simulink model "PlanarModelandControl"  (R2026a)
settings: Solver=FixedStepDiscrete  StopTime=StopTime  FixedStep=Ts  MaxStep=0  RelTol=1e-5  AbsTol=1e-3
PostLoadFcn:
  | Controles_Ativos = 1;
  | Vx_Ref = 0;
  | Vx_init = 0;
  | t_default = [0; 10000];
  | Brake_Cmd = [t_default, [0; 0]];
  | Accel_Cmd = [t_default, [0; 0]];
  | Steer_Cmd = [t_default, [0; 0]];
  | Flag_CruiseControl = 0;
  | Ts = 0.001;
  | load('TC_LUT.mat')
InitFcn:
  | % Carrega parâmetros gerais (Massa, Ganhos PID, Geometria)
  | run('Parameters.m');
  | 
  | % Carrega as Tabelas (Lookup Tables)
  | run('curva_steering.m');
  | run('curva_tq_motor.m');
  | load('TC_LUT.mat')
model workspace (MAT data in the model file):
  A = 1.22
  Ack = 1
  Cd = 1.1
  Cl = -2
  Cs = [19075.58 25992.8 31792.64 35898.02 39044.37]
  Fz0x = -811.9083
  Fz0y = -210.9467
  Fz_cs = [222.41 444.82 667.23 889.64 1112.05]
  IAY = 0
  IAx = 0
  IAy = 0
  Im = 0.00033
  It = 0.0094
  Iw = 0.1578
  Iw_ = 0.7
  Iz_car = 180
  K_50_understeer = 0.005184
  K_Stability = 1
  K_oversteer = -0.001
  Kappa_F = 0
  Kappa_R = 0
  Km_FM = 110000
  Km_Fm = 110000
  Km_RM = 120000
  Km_Rm = 120000
  KphiARB_F = 0
  KphiARB_R = 11000
  KphiF = 17054.5
  KphiR = 40034.8
  Kphim_F = 137500
  Kphim_R = 48600
  L = 1.53
  M = 300
  Mgt = 11.72
  P = 68.9476
  P0 = 55.4852
  R = 0.2032
  Rcurva = 20
  Re = 0.2
  SR = 3
  TV_On = 1
  Ts = 0.001
  W = 2940
  Wf = 308.034
  Wr = 339.426
  Ws = 2099.34
  YLL = 1.11022302463e-16
  cx1 = 1.3668
  cy1 = 1.839
  dpi = 0.242630467224
  dx1 = 2.1568
  dx2 = -0.065814
  dx3 = 0.0011254
  dy1 = 2.453
  dy2 = -0.11197
  dy3 = 10.2899
  ex1 = -0.19815
  ex2 = -0.62529
  ex3 = 1.3396
  ex4 = 0.0029464
  ey1 = 0.96085
  ey2 = -0.28662
  ey3 = 0.14389
  ey4 = 4.9274
  ey5 = -70.7165
  g = 9.81
  hcg = 0.252
  hcgs = 0.202
  hrc_f = 0.056
  hrc_r = 0.084
  hs_f = 0.222
  hs_r = 0.222
  hx1 = -0.003859
  hx2 = -0.000374
  hy1 = -0.000403
  hy2 = -0.000261
  kx1 = -36.9039
  kx2 = 22.3857
  kx3 = 0.61844
  ky1 = -83.2526
  ky2 = -5.565
  ky3 = 0.88491
  ky4 = 2.9994
  ky5 = -99.9393
  ky6 = -3.4705
  ky7 = -0.91452
  lf = 0.8415
  lr = 0.6885
  mass_FL = 67.5
  mass_FR = 67.5
  mass_RL = 82.5
  mass_RR = 82.5
  mi = 2
  ms = 240
  pty1 = 0.005744
  pty2 = 19.1334
  px1 = -0.039964
  px2 = -0.15015
  px3 = -0.32528
  px4 = -0.063273
  py1 = 0.38578
  py2 = 1.2048
  py3 = -0.14544
  py4 = 0.092886
  py5 = -0.82901
  r = 9
  rhoar = 1.22
  s_f = 1
  s_r = 0.9
  tf = 1.25
  tr = 1.25
  vx1 = -0.11922
  vx2 = 0.002205
  vy1 = 0.032907
  vy2 = 0.018068
  vy3 = -1.0429
  vy4 = 0.53712
  x_fl = 0.8415
  x_fr = 0.8415
  x_rl = 0.6885
  x_rr = 0.6885
  xs_f = 0.826
  xs_r = 0.704
  y_fl = 0.6
  y_fr = 0.6
  y_rl = 0.59
  y_rr = 0.59
values from neighbouring files (symbols this model uses):
  GG_mode = 0    [autocross.m]    (also in teste.mat)
  Gr = 11.72    [Parameters.m]    (also in teste.mat)
  K_variavel = [-0.001, 0, 0.0008, 0.0012, 0.0015]    [Parameters.m]    (also in teste.mat = [-0.001 0 0.0008 0.0012 0.0015])
  Ki_data = [283117.9493, 114831.615, 51596.0519, 30556.5344, 17525.221, 11773.8301, 10567.5001]*0.008    [Parameters.m]    (also in teste.mat = [2264.9435944 918.65292 412.7684152 244.4522752 140.201768 9)
  Kp_data = [2877.5702, 2303.9999, 1972.0835, 1556.8775, 1258.2451, 1022.7085, 982.7861, ]*5    [Parameters.m]    (also in teste.mat = [14387.851 11519.9995 9860.4175 7784.3875 6291.2255 5113.542)
  Mz_maxF = [0 66.3 132.6 198.9 265.2 330.23 396.52 462.83 529.112 595.42 661.73 728.03 794.33 860.62 926.92 993.23 1058.25 1124.55 1190.85 1257.15 1323.45 1389.75 1456.05 1522.35 1588.65 1654.95]    [Parameters.m]    (also in teste.mat = [0 66.3 132.6 198.9 265.2 330.23 396.52 462.83 529.112 595.4)
  Mz_minF = [0 -66.3 -132.6 -198.9 -265.2 -330.23 -396.52 -462.83 -529.112 -595.42 -661.73 -728.03 -794.33 -860.62 -926.92 -993.23 -1058.25 -1124.55 -1190.85 -1257.15 -1323.45 -1389.75 -1456.05 -1522.35 -1588.65 -1654.95]    [Parameters.m]    (also in teste.mat = [0 -66.3 -132.6 -198.9 -265.2 -330.23 -396.52 -462.83 -529.1)
  pedal = [0 4 8 12 16 20 24 28 32 36 40 44 48 52 56 60 64 68 72 76 80 84 88 92 96 100]    [Parameters.m]    (also in teste.mat = [0 4 8 12 16 20 24 28 32 36 40 44 ... (26 elements, 1x26)])
  rpm_355_novo = [0 10050 10100 10140 10170 10210 10250 10280 10320 10360         10390 10420 10460 10620 10770 10920 11050 11170 11280         11380 11470 11550 11625 11680 11730 11775 11790 11800]    [curva_tq_motor.m]    (also in teste.mat = [0 10050 10100 10140 10170 10210 10250 10280 10320 10360 103)
  Steering_inner = [-29.018 ... (first row only)    [curva_steering.m]    (also in teste.mat = [-29.018 -25.595 -22.357 -19.265 -16.292 -13.415 -10.619 -7.)
  Steering_outer = [-25.111 ... (first row only)    [curva_steering.m]    (also in teste.mat = [-25.111 -22.589 -20.082 -17.585 -15.094 -12.604 -10.11 -7.6)
  steering_vol = [-105 ... (first row only)    [curva_steering.m]    (also in teste.mat = [-105 -94.5 -84 -73.5 -63 -52.5 -42 -31.5 -21 -10.5 0 10.5 .)
  torque_355_novo = [16.2 16.2 16.0 15.8 15.6 15.4 15.2 15.0 14.8 14.6            14.4 14.2 14.0 13.0 12.0 11.0 10.0 9.0 8.0            7.0 6.0 5.0 4.0 3.0 2.0 1.0 0.5 0.0]    [curva_tq_motor.m]    (also in teste.mat = [16.2 16.2 16 15.8 15.6 15.4 15.2 15 14.8 14.6 14.4 14.2 ...)
  Vx = [0, 12.5, 23.6, 30, 35]    [Parameters.m]    (also in teste.mat = [0 12.5 23.6 30 35])
  Vx_axis = [5, 10, 15, 20, 25, 30, 35]    [Parameters.m]    (also in teste.mat = [5 10 15 20 25 30 35])
undefined symbols (not defined in the file or in its folder): DiscIntNeverNeededParam, magnitude
libraries: simulink x5, fuzblock x4, sl_discontinuities x3, simulink_extras x3, slpidlib x1
(part 3 of 4)

== system PlanarModelandControl/Planta/Dinamica do Veículo/Load Transfer/Longitudinal Weight Transfer ==
  note: Transferencia de Carga - Longitudinal
  Bus Element In4 : Inport  PortName=Movimento  Element=Ax_cg
  Discrete Transfer Fcn1 : DiscreteTransferFcn  Numerator=[0.1813]  Denominator="[1  -0.8187]"
  Gain21 : Gain  Gain=-1/2*W*(1-2*YLL/tf)*(hcg/(L*g))
  Gain22 : Gain  Gain=1/2*W*hcg*(1-2*YLL/tr)/(L*g)
  Gain23 : Gain  Gain=1/2*W*hcg*(1+2*YLL/tr)/(L*g)
  Gain24 : Gain  Gain=-1/2*W*(1+2*YLL/tf)*(hcg/(L*g))
  Out Bus Element : Outport  PortName=DeltaW_long  Element=DeltaW_FL
  Out Bus Element1 : Outport  PortName=DeltaW_long  Element=DeltaW_FR
  Out Bus Element2 : Outport  PortName=DeltaW_long  Element=DeltaW_RL
  Out Bus Element3 : Outport  PortName=DeltaW_long  Element=DeltaW_RR
  wires:
  Bus Element In4/1 -> Discrete Transfer Fcn1/1
  Discrete Transfer Fcn1/1 -> Gain24/1, Gain23/1, Gain22/1, Gain21/1
  Gain21/1 -> Out Bus Element/1
  Gain24/1 -> Out Bus Element1/1
  Gain22/1 -> Out Bus Element2/1
  Gain23/1 -> Out Bus Element3/1

== system PlanarModelandControl/Planta/Dinamica do Veículo/Load Transfer/Total Normal Forces ==
  FLift : Inport
  BusElementIn : Inport  Port=2  PortName=DeltaW_long  Element=DeltaW_RR
  BusElementIn1 : Inport  Port=2  PortName=DeltaW_long  Element=DeltaW_RL
  BusElementIn2 : Inport  Port=2  PortName=DeltaW_long  Element=DeltaW_FL
  BusElementIn3 : Inport  Port=2  PortName=DeltaW_long  Element=DeltaW_FR
  BusElementIn4 : Inport  Port=3  PortName=DeltaW_lat  Element=WLR_FL
  BusElementIn5 : Inport  Port=3  PortName=DeltaW_lat  Element=WLR_FR
  BusElementIn6 : Inport  Port=3  PortName=DeltaW_lat  Element=WLR_RL
  BusElementIn7 : Inport  Port=3  PortName=DeltaW_lat  Element=WLR_RR
  Constant20 : Constant  Value=mass_FL*g
  Constant21 : Constant  Value=mass_RL*g
  Constant22 : Constant  Value=mass_FR*g
  Constant23 : Constant  Value=mass_RR*g
  Gain35 : Gain  Gain=0.15
  Gain36 : Gain  Gain=0.35
  Gain37 : Gain  Gain=-1
  Gain38 : Gain  Gain=-1
  Gain8 : Gain  Gain=0.35
  Gain9 : Gain  Gain=0.15
  MATLAB Function4 : MATLAB Function  TreatAsAtomicUnit=on
  MATLAB Function5 : MATLAB Function  TreatAsAtomicUnit=on
  Sum{20..23} (4 alike) : Sum  Inputs=|++++
  Bus Element Out11 : Outport  VectorParamsAs1DForOutWhenUnconnected=off  PortName=NormalForces  Element=Fz_FL
  Bus Element Out12 : Outport  VectorParamsAs1DForOutWhenUnconnected=off  PortName=NormalForces  Element=Fz_RL
  Bus Element Out13 : Outport  VectorParamsAs1DForOutWhenUnconnected=off  PortName=NormalForces  Element=Fz_RR
  Bus Element Out14 : Outport  VectorParamsAs1DForOutWhenUnconnected=off  PortName=NormalForces  Element=Fz_FR
  wires:
  Gain35/1 -> Sum23/3  "Lift"
  Sum21/1 -> MATLAB Function5/1(u)  "FR"
  Constant22/1 -> Sum21/4
  Gain9/1 -> Sum22/3  "lift"
  Constant20/1 -> Sum20/4
  BusElementIn4/1 -> Gain37/1
  MATLAB Function5/1(y) -> Bus Element Out14/1
  Gain8/1 -> Sum20/3  "Lift"
  Sum23/1 -> Bus Element Out13/1  "RR"
  BusElementIn7/1 -> Sum23/2  "Lateral"
  FLift/1 -> Gain8/1, Gain35/1, Gain36/1, Gain9/1
  Constant23/1 -> Sum23/4  "Peso"
  Gain38/1 -> Sum22/2
  BusElementIn1/1 -> Sum22/1
  BusElementIn6/1 -> Gain38/1
  BusElementIn5/1 -> Sum21/2
  MATLAB Function4/1(y) -> Bus Element Out11/1  "Fz"
  Gain37/1 -> Sum20/2
  Sum20/1 -> MATLAB Function4/1(u)  "FL"
  Constant21/1 -> Sum22/4  "peso"
  Gain36/1 -> Sum21/3
  BusElementIn3/1 -> Sum21/1
  BusElementIn2/1 -> Sum20/1
  Sum22/1 -> Bus Element Out12/1  "RL"
  BusElementIn/1 -> Sum23/1

== system PlanarModelandControl/Planta/Dinamica do Veículo/Pacejka/Pacejka Lat FL1 ==
  Bus Element In2 : Inport  PortName=SAs
  Bus Element In{1,3..8} (7 alike) : Inport  Port=2  PortName=Fz
  In Bus Element : Inport  Port=3  PortName=SA
  By : Subsystem
  Constant : Constant  Value=cy1  SampleTime=0.01
  Dy : Subsystem
  Ey : Subsystem
  KyIA : Subsystem
  KySA : Subsystem
  MATLAB Function : MATLAB Function  TreatAsAtomicUnit=on
  SAy : Subsystem
  SHy : Subsystem
  SVy : Subsystem
      settings: PortSchema="{\"entries\":[{\"content\":{\"sides\":[{\"content\":{\"connectorIds\":[\"In1\",\"In2\"]},\"type\":\"ConnectorPlacement.EquallySpacedRectSide\",\"uuid\":\"4dd0695f-05b0-48b0-a9fc-081fec962ad7\"},{\"content\":{\"connectorIds\":[\"Out1\"],\"side\":\"RIGHT\"},\"type\":\"ConnectorPlacement.EquallySpacedRectSide\",\"uuid\":\"f276d9c5-1b17-4b74-902d-99702de63a48\"},{\"content\":{\"side\":\"TOP\"},\"type\":\"ConnectorPlacement.EquallySpacedRectSide\",\"uuid\":\"e4f1334c-9257-4c80-bdfa-4e6196575c00\"},{\"content\":{\"connectorIds\":[\"In3\"],\"side\":\"BOTTOM\"},\"type\":\"ConnectorPlacement.EquallySpacedRectSide\",\"uuid\":\"070f7b12-16f3-4448-8e7e-3456eb7f9f12\"}]},\"type\":\"ConnectorPlacement.EquallySpacedConnector\",\"uuid\":\"55c720f1-612b-48de-a64e-292835aa1348\"}],\"packageUris\":[\"http://schema.mathworks.com/mf0/Connector/R2020b\"],\"version\":\"1.0\"}"
  SVyIA : Subsystem
  dfzy : Subsystem
  Fy0 : Outport
  wires:
  SVy/1(SVy) -> MATLAB Function/6(SVy)  "SVy"
  Ey/1(Ey) -> MATLAB Function/5(Ey)  "ey"
  SAy/1(SAy) -> Ey/1(SAy), MATLAB Function/7(SAy)  "SAy"
  Dy/1(Dy) -> By/2(Dy), MATLAB Function/2(Dy)
  Constant/1 -> MATLAB Function/3(Cy), By/3(Cy)  "cy"
  By/1(By) -> MATLAB Function/4(By)  "by"
  MATLAB Function/1(Fy) -> Fy0/1
  Bus Element In1/1 -> MATLAB Function/1(Fz)
  Bus Element In2/1 -> SAy/1(SAs)
  Bus Element In3/1 -> dfzy/1(Fz)
  Bus Element In4/1 -> KySA/1(Fz)
  Bus Element In5/1 -> KyIA/1(Fz)
  Bus Element In6/1 -> SVyIA/1(Fz)
  SHy/1(SHy) -> SAy/2(SHy)
  dfzy/1(dfzy) -> [dfzy]
  [dfzy] -> SVyIA/2(dfzy)
  [dfzy] -> SHy/4(dfzy)
  [dfzy] -> SVy/2(dfzy)
  [dfzy] -> Dy/2(dfzy)
  Bus Element In7/1 -> Dy/1(Fz)
  Bus Element In8/1 -> SVy/1(Fz)
  [dfzy] -> Ey/2(dfzy)
  SVyIA/1(SVylA) -> SVy/3(SVylA), SHy/3(SVylA)
  [dfzy] -> KyIA/2(dfzy)
  KyIA/1(KylA) -> SHy/2(KylA)
  KySA/1(KySA) -> By/1(KySA), SHy/1(KySA)
  In Bus Element/1 -> MATLAB Function/8(SA)

== system PlanarModelandControl/Planta/Dinamica do Veículo/Pacejka/Pacejka Lat FL2 ==
  Bus Element In2 : Inport  PortName=SAs
  Bus Element In{1,3..8} (7 alike) : Inport  Port=2  PortName=Fz
  In Bus Element : Inport  Port=3  PortName=SA
  By : Subsystem
  Constant : Constant  Value=cy1  SampleTime=0.01
  Dy : Subsystem
  Ey : Subsystem
  KyIA : Subsystem
  KySA : Subsystem
  MATLAB Function : MATLAB Function  TreatAsAtomicUnit=on
  SAy : Subsystem
  SHy : Subsystem
  SVy : Subsystem
      settings: PortSchema="{\"entries\":[{\"content\":{\"sides\":[{\"content\":{\"connectorIds\":[\"In1\",\"In2\"]},\"type\":\"ConnectorPlacement.EquallySpacedRectSide\",\"uuid\":\"4dd0695f-05b0-48b0-a9fc-081fec962ad7\"},{\"content\":{\"connectorIds\":[\"Out1\"],\"side\":\"RIGHT\"},\"type\":\"ConnectorPlacement.EquallySpacedRectSide\",\"uuid\":\"f276d9c5-1b17-4b74-902d-99702de63a48\"},{\"content\":{\"side\":\"TOP\"},\"type\":\"ConnectorPlacement.EquallySpacedRectSide\",\"uuid\":\"e4f1334c-9257-4c80-bdfa-4e6196575c00\"},{\"content\":{\"connectorIds\":[\"In3\"],\"side\":\"BOTTOM\"},\"type\":\"ConnectorPlacement.EquallySpacedRectSide\",\"uuid\":\"070f7b12-16f3-4448-8e7e-3456eb7f9f12\"}]},\"type\":\"ConnectorPlacement.EquallySpacedConnector\",\"uuid\":\"55c720f1-612b-48de-a64e-292835aa1348\"}],\"packageUris\":[\"http://schema.mathworks.com/mf0/Connector/R2020b\"],\"version\":\"1.0\"}"
  SVyIA : Subsystem
  dfzy : Subsystem
  Fy0 : Outport
  wires:
  SVy/1(SVy) -> MATLAB Function/6(SVy)  "SVy"
  Ey/1(Ey) -> MATLAB Function/5(Ey)  "ey"
  SAy/1(SAy) -> Ey/1(SAy), MATLAB Function/7(SAy)  "SAy"
  Dy/1(Dy) -> By/2(Dy), MATLAB Function/2(Dy)
  Constant/1 -> MATLAB Function/3(Cy), By/3(Cy)  "cy"
  By/1(By) -> MATLAB Function/4(By)  "by"
  MATLAB Function/1(Fy) -> Fy0/1
  Bus Element In1/1 -> MATLAB Function/1(Fz)
  Bus Element In2/1 -> SAy/1(SAs)
  Bus Element In3/1 -> dfzy/1(Fz)
  Bus Element In4/1 -> KySA/1(Fz)
  Bus Element In5/1 -> KyIA/1(Fz)
  Bus Element In6/1 -> SVyIA/1(Fz)
  SHy/1(SHy) -> SAy/2(SHy)
  dfzy/1(dfzy) -> [dfzy]
  [dfzy] -> SVyIA/2(dfzy)
  [dfzy] -> SHy/4(dfzy)
  [dfzy] -> SVy/2(dfzy)
  [dfzy] -> Dy/2(dfzy)
  Bus Element In7/1 -> Dy/1(Fz)
  Bus Element In8/1 -> SVy/1(Fz)
  [dfzy] -> Ey/2(dfzy)
  SVyIA/1(SVylA) -> SVy/3(SVylA), SHy/3(SVylA)
  [dfzy] -> KyIA/2(dfzy)
  KyIA/1(KylA) -> SHy/2(KylA)
  KySA/1(KySA) -> By/1(KySA), SHy/1(KySA)
  In Bus Element/1 -> MATLAB Function/8(SA)

== system PlanarModelandControl/Planta/Dinamica do Veículo/Pacejka/Pacejka Lat FL3 ==
  Bus Element In2 : Inport  PortName=SAs
  Bus Element In{1,3..8} (7 alike) : Inport  Port=2  PortName=Fz
  In Bus Element : Inport  Port=3  PortName=SA
  By : Subsystem
  Constant : Constant  Value=cy1  SampleTime=0.01
  Dy : Subsystem
  Ey : Subsystem
  KyIA : Subsystem
  KySA : Subsystem
  MATLAB Function : MATLAB Function  TreatAsAtomicUnit=on
  SAy : Subsystem
  SHy : Subsystem
  SVy : Subsystem
      settings: PortSchema="{\"entries\":[{\"content\":{\"sides\":[{\"content\":{\"connectorIds\":[\"In1\",\"In2\"]},\"type\":\"ConnectorPlacement.EquallySpacedRectSide\",\"uuid\":\"4dd0695f-05b0-48b0-a9fc-081fec962ad7\"},{\"content\":{\"connectorIds\":[\"Out1\"],\"side\":\"RIGHT\"},\"type\":\"ConnectorPlacement.EquallySpacedRectSide\",\"uuid\":\"f276d9c5-1b17-4b74-902d-99702de63a48\"},{\"content\":{\"side\":\"TOP\"},\"type\":\"ConnectorPlacement.EquallySpacedRectSide\",\"uuid\":\"e4f1334c-9257-4c80-bdfa-4e6196575c00\"},{\"content\":{\"connectorIds\":[\"In3\"],\"side\":\"BOTTOM\"},\"type\":\"ConnectorPlacement.EquallySpacedRectSide\",\"uuid\":\"070f7b12-16f3-4448-8e7e-3456eb7f9f12\"}]},\"type\":\"ConnectorPlacement.EquallySpacedConnector\",\"uuid\":\"55c720f1-612b-48de-a64e-292835aa1348\"}],\"packageUris\":[\"http://schema.mathworks.com/mf0/Connector/R2020b\"],\"version\":\"1.0\"}"
  SVyIA : Subsystem
  dfzy : Subsystem
  Fy0 : Outport
  wires:
  SVy/1(SVy) -> MATLAB Function/6(SVy)  "SVy"
  Ey/1(Ey) -> MATLAB Function/5(Ey)  "ey"
  SAy/1(SAy) -> Ey/1(SAy), MATLAB Function/7(SAy)  "SAy"
  Dy/1(Dy) -> By/2(Dy), MATLAB Function/2(Dy)
  Constant/1 -> MATLAB Function/3(Cy), By/3(Cy)  "cy"
  By/1(By) -> MATLAB Function/4(By)  "by"
  MATLAB Function/1(Fy) -> Fy0/1
  Bus Element In1/1 -> MATLAB Function/1(Fz)
  Bus Element In2/1 -> SAy/1(SAs)
  Bus Element In3/1 -> dfzy/1(Fz)
  Bus Element In4/1 -> KySA/1(Fz)
  Bus Element In5/1 -> KyIA/1(Fz)
  Bus Element In6/1 -> SVyIA/1(Fz)
  SHy/1(SHy) -> SAy/2(SHy)
  dfzy/1(dfzy) -> [dfzy]
  [dfzy] -> SVyIA/2(dfzy)
  [dfzy] -> SHy/4(dfzy)
  [dfzy] -> SVy/2(dfzy)
  [dfzy] -> Dy/2(dfzy)
  Bus Element In7/1 -> Dy/1(Fz)
  Bus Element In8/1 -> SVy/1(Fz)
  [dfzy] -> Ey/2(dfzy)
  SVyIA/1(SVylA) -> SVy/3(SVylA), SHy/3(SVylA)
  [dfzy] -> KyIA/2(dfzy)
  KyIA/1(KylA) -> SHy/2(KylA)
  KySA/1(KySA) -> By/1(KySA), SHy/1(KySA)
  In Bus Element/1 -> MATLAB Function/8(SA)

== system PlanarModelandControl/Planta/Dinamica do Veículo/Pacejka/Pacejka Lat FL4 ==
  Bus Element In2 : Inport  PortName=SAs
  Bus Element In{1,3..8} (7 alike) : Inport  Port=2  PortName=Fz
  In Bus Element : Inport  Port=3  PortName=SA
  By : Subsystem
  Constant : Constant  Value=cy1  SampleTime=0.01
  Dy : Subsystem
  Ey : Subsystem
  KyIA : Subsystem
  KySA : Subsystem
  MATLAB Function : MATLAB Function  TreatAsAtomicUnit=on
  SAy : Subsystem
  SHy : Subsystem
  SVy : Subsystem
      settings: PortSchema="{\"entries\":[{\"content\":{\"sides\":[{\"content\":{\"connectorIds\":[\"In1\",\"In2\"]},\"type\":\"ConnectorPlacement.EquallySpacedRectSide\",\"uuid\":\"4dd0695f-05b0-48b0-a9fc-081fec962ad7\"},{\"content\":{\"connectorIds\":[\"Out1\"],\"side\":\"RIGHT\"},\"type\":\"ConnectorPlacement.EquallySpacedRectSide\",\"uuid\":\"f276d9c5-1b17-4b74-902d-99702de63a48\"},{\"content\":{\"side\":\"TOP\"},\"type\":\"ConnectorPlacement.EquallySpacedRectSide\",\"uuid\":\"e4f1334c-9257-4c80-bdfa-4e6196575c00\"},{\"content\":{\"connectorIds\":[\"In3\"],\"side\":\"BOTTOM\"},\"type\":\"ConnectorPlacement.EquallySpacedRectSide\",\"uuid\":\"070f7b12-16f3-4448-8e7e-3456eb7f9f12\"}]},\"type\":\"ConnectorPlacement.EquallySpacedConnector\",\"uuid\":\"55c720f1-612b-48de-a64e-292835aa1348\"}],\"packageUris\":[\"http://schema.mathworks.com/mf0/Connector/R2020b\"],\"version\":\"1.0\"}"
  SVyIA : Subsystem
  dfzy : Subsystem
  Fy0 : Outport
  wires:
  SVy/1(SVy) -> MATLAB Function/6(SVy)  "SVy"
  Ey/1(Ey) -> MATLAB Function/5(Ey)  "ey"
  SAy/1(SAy) -> Ey/1(SAy), MATLAB Function/7(SAy)  "SAy"
  Dy/1(Dy) -> By/2(Dy), MATLAB Function/2(Dy)
  Constant/1 -> MATLAB Function/3(Cy), By/3(Cy)  "cy"
  By/1(By) -> MATLAB Function/4(By)  "by"
  MATLAB Function/1(Fy) -> Fy0/1
  Bus Element In1/1 -> MATLAB Function/1(Fz)
  Bus Element In2/1 -> SAy/1(SAs)
  Bus Element In3/1 -> dfzy/1(Fz)
  Bus Element In4/1 -> KySA/1(Fz)
  Bus Element In5/1 -> KyIA/1(Fz)
  Bus Element In6/1 -> SVyIA/1(Fz)
  SHy/1(SHy) -> SAy/2(SHy)
  dfzy/1(dfzy) -> [dfzy]
  [dfzy] -> SVyIA/2(dfzy)
  [dfzy] -> SHy/4(dfzy)
  [dfzy] -> SVy/2(dfzy)
  [dfzy] -> Dy/2(dfzy)
  Bus Element In7/1 -> Dy/1(Fz)
  Bus Element In8/1 -> SVy/1(Fz)
  [dfzy] -> Ey/2(dfzy)
  SVyIA/1(SVylA) -> SVy/3(SVylA), SHy/3(SVylA)
  [dfzy] -> KyIA/2(dfzy)
  KyIA/1(KylA) -> SHy/2(KylA)
  KySA/1(KySA) -> By/1(KySA), SHy/1(KySA)
  In Bus Element/1 -> MATLAB Function/8(SA)

== system PlanarModelandControl/Planta/Dinamica do Veículo/Pacejka/Pacejka Long FL  ==
  note: Longitudinal
  Bus Element In1 : Inport  PortName=SL
  Bus Element In2 : Inport  PortName=SL
  Bus Element In{3..6} (4 alike) : Inport  Port=2  PortName=Fz
  Bx : Subsystem
  Constant : Constant  Value=0
  Constant1 : Constant  Value=cx1  SampleTime=0.01
  Dx : Subsystem
  Ex : Subsystem
  Kx : Subsystem
  MATLAB Function : MATLAB Function  TreatAsAtomicUnit=on
  Slip : Subsystem
  Svx : Subsystem
  Switch : Switch  Criteria=u2 ~= 0  SaturateOnIntegerOverflow=off  InputSameDT=off
  dfz : Subsystem
  Fx0 : Outport
  Kx_out : Outport  Port=2
  Dx_out : Outport  Port=3
  wires:
  Bx/1(Bx) -> MATLAB Function/4(Bx)  "Bx"
  Ex/1(Ex) -> MATLAB Function/5(Ex)  "Ex"
  Constant1/1 -> MATLAB Function/3(Cx)  "Cx"
  Svx/1(Svx) -> MATLAB Function/6(SVx)  "Svx"
  Dx/1(Dx) -> Dx_out/1, MATLAB Function/2(Dx)  "Dx"
  Slip/1("Slx ") -> MATLAB Function/1(Slx)
  MATLAB Function/1(Fx) -> Switch/1  "Fx"
  Constant/1 -> Switch/3
  Switch/1 -> Fx0/1  "Fx"
  Bus Element In2/1 -> Switch/2
  Bus Element In3/1 -> Dx/2(Fz)
  Bus Element In4/1 -> dfz/1(Fz)
  Bus Element In5/1 -> Kx/1(Fz)
  Bus Element In6/1 -> Svx/2(Fz)
  Bus Element In1/1 -> Ex/2(SL), Slip/2(SL)
  dfz/1(Dfz) -> Ex/1(Dfz), Svx/1(Dfz), Bx/2(Dx), Dx/1(Dfz), Kx/2(Dfz), Slip/1(Dfz)
  Kx/1(Kx) -> Kx_out/1, Bx/1(Kx)

== system PlanarModelandControl/Planta/Dinamica do Veículo/Pacejka/Pacejka Long FR ==
  note: Longitudinal
  Bus Element In1 : Inport  PortName=SL
  Bus Element In2 : Inport  PortName=SL
  Bus Element In{3..6} (4 alike) : Inport  Port=2  PortName=Fz
  Bx : Subsystem
  Constant : Constant  Value=0
  Constant1 : Constant  Value=cx1  SampleTime=0.01
  Dx : Subsystem
  Ex : Subsystem
  Kx : Subsystem
  MATLAB Function : MATLAB Function  TreatAsAtomicUnit=on
  Slip : Subsystem
  Svx : Subsystem
  Switch : Switch  Criteria=u2 ~= 0  SaturateOnIntegerOverflow=off  InputSameDT=off
  dfz : Subsystem
  Fx0 : Outport
  Kx_out : Outport  Port=2
  Dx_out : Outport  Port=3
  wires:
  Bx/1(Bx) -> MATLAB Function/4(Bx)  "Bx"
  Ex/1(Ex) -> MATLAB Function/5(Ex)  "Ex"
  Constant1/1 -> MATLAB Function/3(Cx)  "Cx"
  Svx/1(Svx) -> MATLAB Function/6(SVx)  "Svx"
  Dx/1(Dx) -> Dx_out/1, MATLAB Function/2(Dx)  "Dx"
  Slip/1("Slx ") -> MATLAB Function/1(Slx)
  MATLAB Function/1(Fx) -> Switch/1  "Fx"
  Constant/1 -> Switch/3
  Switch/1 -> Fx0/1  "Fx"
  Bus Element In2/1 -> Switch/2
  Bus Element In3/1 -> Dx/2(Fz)
  Bus Element In4/1 -> dfz/1(Fz)
  Bus Element In5/1 -> Kx/1(Fz)
  Bus Element In6/1 -> Svx/2(Fz)
  Bus Element In1/1 -> Ex/2(SL), Slip/2(SL)
  dfz/1(Dfz) -> Ex/1(Dfz), Svx/1(Dfz), Bx/2(Dx), Dx/1(Dfz), Kx/2(Dfz), Slip/1(Dfz)
  Kx/1(Kx) -> Kx_out/1, Bx/1(Kx)

== system PlanarModelandControl/Planta/Dinamica do Veículo/Pacejka/Pacejka Long RL ==
  note: Longitudinal
  Bus Element In1 : Inport  PortName=SL
  Bus Element In2 : Inport  PortName=SL
  Bus Element In{3..6} (4 alike) : Inport  Port=2  PortName=Fz
  Bx : Subsystem
  Constant : Constant  Value=0
  Constant1 : Constant  Value=cx1  SampleTime=0.01
  Dx : Subsystem
  Ex : Subsystem
  Kx : Subsystem
  MATLAB Function : MATLAB Function  TreatAsAtomicUnit=on
  Slip : Subsystem
  Svx : Subsystem
  Switch : Switch  Criteria=u2 ~= 0  SaturateOnIntegerOverflow=off  InputSameDT=off
  dfz : Subsystem
  Fx0 : Outport
  Kx_out : Outport  Port=2
  Dx_out : Outport  Port=3
  wires:
  Bx/1(Bx) -> MATLAB Function/4(Bx)  "Bx"
  Ex/1(Ex) -> MATLAB Function/5(Ex)  "Ex"
  Constant1/1 -> MATLAB Function/3(Cx)  "Cx"
  Svx/1(Svx) -> MATLAB Function/6(SVx)  "Svx"
  Dx/1(Dx) -> Dx_out/1, MATLAB Function/2(Dx)  "Dx"
  Slip/1("Slx ") -> MATLAB Function/1(Slx)
  MATLAB Function/1(Fx) -> Switch/1  "Fx"
  Constant/1 -> Switch/3
  Switch/1 -> Fx0/1  "Fx"
  Bus Element In2/1 -> Switch/2
  Bus Element In3/1 -> Dx/2(Fz)
  Bus Element In4/1 -> dfz/1(Fz)
  Bus Element In5/1 -> Kx/1(Fz)
  Bus Element In6/1 -> Svx/2(Fz)
  Bus Element In1/1 -> Ex/2(SL), Slip/2(SL)
  dfz/1(Dfz) -> Ex/1(Dfz), Svx/1(Dfz), Bx/2(Dx), Dx/1(Dfz), Kx/2(Dfz), Slip/1(Dfz)
  Kx/1(Kx) -> Kx_out/1, Bx/1(Kx)

== system PlanarModelandControl/Planta/Dinamica do Veículo/Pacejka/Pacejka Long RR ==
  note: Longitudinal
  Bus Element In1 : Inport  PortName=SL
  Bus Element In2 : Inport  PortName=SL
  Bus Element In{3..6} (4 alike) : Inport  Port=2  PortName=Fz
  Bx : Subsystem
  Constant : Constant  Value=0
  Constant1 : Constant  Value=cx1  SampleTime=0.01
  Dx : Subsystem
  Ex : Subsystem
  Kx : Subsystem
  MATLAB Function : MATLAB Function  TreatAsAtomicUnit=on
  Slip : Subsystem
  Svx : Subsystem
  Switch : Switch  Criteria=u2 ~= 0  SaturateOnIntegerOverflow=off  InputSameDT=off
  dfz : Subsystem
  Fx0 : Outport
  Kx_out : Outport  Port=2
  Dx_out : Outport  Port=3
  wires:
  Bx/1(Bx) -> MATLAB Function/4(Bx)  "Bx"
  Ex/1(Ex) -> MATLAB Function/5(Ex)  "Ex"
  Constant1/1 -> MATLAB Function/3(Cx)  "Cx"
  Svx/1(Svx) -> MATLAB Function/6(SVx)  "Svx"
  Dx/1(Dx) -> Dx_out/1, MATLAB Function/2(Dx)  "Dx"
  Slip/1("Slx ") -> MATLAB Function/1(Slx)
  MATLAB Function/1(Fx) -> Switch/1  "Fx"
  Constant/1 -> Switch/3
  Switch/1 -> Fx0/1  "Fx"
  Bus Element In2/1 -> Switch/2
  Bus Element In3/1 -> Dx/2(Fz)
  Bus Element In4/1 -> dfz/1(Fz)
  Bus Element In5/1 -> Kx/1(Fz)
  Bus Element In6/1 -> Svx/2(Fz)
  Bus Element In1/1 -> Ex/2(SL), Slip/2(SL)
  dfz/1(Dfz) -> Ex/1(Dfz), Svx/1(Dfz), Bx/2(Dx), Dx/1(Dfz), Kx/2(Dfz), Slip/1(Dfz)
  Kx/1(Kx) -> Kx_out/1, Bx/1(Kx)
